simulink model "Poly_creator"  (R2022a)
settings: Solver=FixedStepAuto  StartTime=0.0  StopTime=10  FixedStep=1e-3  RelTol=1e-3
values from neighbouring files (symbols this model uses):
  A = 0.0001    [parameterSetting.m]
  base = Mb*9.8/4+mw*9.8+0.5*9.8*Mv    (= 46305)    [parameterSetting.m]
  d = 2.6    [parameterSetting.m]
  I = 3055e-8    (= 3.055e-05)    [parameterSetting.m]
  Jv = 3044    [parameterSetting.m]
  kf = 6.67e7    (= 66700000)    [parameterSetting.m]
  Kv = 1.5e6    (= 1500000)    [parameterSetting.m]
  L = beamLength(k)*210    [BuildDataSample.m]    (also in parameterSetting.m = 210)
  m = 7800*(7684e-6)    (= 59.9352)    [parameterSetting.m]
  Mv = 4060/2    (= 2030)    [parameterSetting.m]
  mw = 1670/2    (= 835)    [parameterSetting.m]
  N1 = 0    [DatacreatorForChapter4.m]
  N2 = 0    [DatacreatorForChapter4.m]
  position = 0.55*L    [DatacreatorForChapter4.m]    (also in Figure4_9.m = 94.5; chapter4_3.m = 105; parameterSetting.m = 105)
  r = 0.25    [chapter4_3.m]    (also in parameterSetting.m = 0.42)
  v = vkmh/3.6    [BuildDataSample.m]    (also in DatacreatorForChapter4.m = vkmh_set(j)/3.6; Figure4_9.m = vkmh_set(i); chapter4_1.m = vkmh_set(i)/3.6; chapter4_3.m = 13.8888888889)
(part 2 of 79)

== system Poly_creator/Subsystem3/Subsystem1/Subsystem4 ==
  In1 : Inport
  In2 : Inport  Port=2
  Add : Sum  Inputs=++++++++++++++++++++
  Add Constant{,1..18} (19 alike) : Bias  Bias=1  SaturateOnIntegerOverflow=off
  Subsystem1 : Subsystem
  Subsystem10 : Subsystem
  Subsystem11 : Subsystem
  Subsystem12 : Subsystem
  Subsystem13 : Subsystem
  Subsystem14 : Subsystem
  Subsystem15 : Subsystem
  Subsystem16 : Subsystem
  Subsystem17 : Subsystem
  Subsystem18 : Subsystem
  Subsystem19 : Subsystem
  Subsystem2 : Subsystem
  Subsystem20 : Subsystem
  Subsystem3 : Subsystem
  Subsystem4 : Subsystem
  Subsystem5 : Subsystem
  Subsystem6 : Subsystem
  Subsystem7 : Subsystem
  Subsystem8 : Subsystem
  Subsystem9 : Subsystem
  Out1 : Outport
  wires:
  Add Constant7/1 -> Subsystem13/1(index), Add Constant8/1
  Subsystem15/1(Out1) -> Add/15
  [Ff] -> Subsystem3/2(F)
  Add Constant14/1 -> Subsystem12/1(index), Add Constant15/1
  Subsystem8/1(Out1) -> Add/5
  [Ff] -> Subsystem7/2(F)
  Add Constant8/1 -> Subsystem9/1(index), Add Constant9/1
  Subsystem9/1(Out1) -> Add/10
  [Ff] -> Subsystem6/2(F)
  Add Constant4/1 -> Subsystem4/1(index), Add Constant5/1
  Subsystem18/1(Out1) -> Add/20
  Add Constant15/1 -> Subsystem15/1(index), Add Constant16/1
  Subsystem4/1(Out1) -> Add/6
  Add Constant6/1 -> Subsystem6/1(index), Add Constant7/1
  Subsystem16/1(Out1) -> Add/13
  In1/1 -> Add Constant/1, Subsystem5/1(index)
  Subsystem20/1(Out1) -> Add/17
  [Ff] -> Subsystem17/2(F)
  [Ff] -> Subsystem10/2(F)
  Add Constant10/1 -> Subsystem10/1(index), Add Constant11/1
  Add Constant12/1 -> Subsystem17/1(index), Add Constant13/1
  Add Constant3/1 -> Subsystem8/1(index), Add Constant4/1
  Add Constant/1 -> Subsystem1/1(index), Add Constant1/1
  [Ff] -> Subsystem11/2(F)
  Subsystem11/1(Out1) -> Add/11
  [Ff] -> Subsystem19/2(F)
  Subsystem5/1(Out1) -> Add/1
  [Ff] -> Subsystem15/2(F)
  Subsystem1/1(Out1) -> Add/2
  Add Constant13/1 -> Subsystem19/1(index), Add Constant18/1
  [Ff] -> Subsystem9/2(F)
  Add Constant1/1 -> Subsystem3/1(index), Add Constant2/1
  Subsystem10/1(Out1) -> Add/12
  Add Constant2/1 -> Subsystem2/1(index), Add Constant3/1
  [Ff] -> Subsystem2/2(F)
  Subsystem12/1(Out1) -> Add/14
  [Ff] -> Subsystem18/2(F)
  [Ff] -> Subsystem4/2(F)
  Subsystem17/1(Out1) -> Add/18
  Subsystem13/1(Out1) -> Add/9
  [Ff] -> Subsystem1/2(F)
  [Ff] -> Subsystem16/2(F)
  Subsystem14/1(Out1) -> Add/16
  [Ff] -> Subsystem5/2(F)
  [Ff] -> Subsystem20/2(F)
  Subsystem3/1(Out1) -> Add/3
  Subsystem7/1(Out1) -> Add/7
  Add/1 -> Out1/1
  [Ff] -> Subsystem14/2(F)
  [Ff] -> Subsystem8/2(F)
  Subsystem19/1(Out1) -> Add/19
  [Ff] -> Subsystem12/2(F)
  [Ff] -> Subsystem13/2(F)
  Add Constant9/1 -> Subsystem11/1(index), Add Constant10/1
  Add Constant5/1 -> Subsystem7/1(index), Add Constant6/1
  Subsystem6/1(Out1) -> Add/8
  Add Constant11/1 -> Subsystem16/1(index), Add Constant14/1
  Subsystem2/1(Out1) -> Add/4
  Add Constant16/1 -> Subsystem14/1(index), Add Constant17/1
  Add Constant17/1 -> Subsystem20/1(index), Add Constant12/1
  Add Constant18/1 -> Subsystem18/1(index)
  In2/1 -> [Ff]

== system Poly_creator/Subsystem3/Subsystem1/Subsystem5 ==
  In1 : Inport
  In2 : Inport  Port=2
  Add : Sum  Inputs=++++++++++++++++++++
  Add Constant{,1..18} (19 alike) : Bias  Bias=1  SaturateOnIntegerOverflow=off
  Subsystem1 : Subsystem
  Subsystem10 : Subsystem
  Subsystem11 : Subsystem
  Subsystem12 : Subsystem
  Subsystem13 : Subsystem
  Subsystem14 : Subsystem
  Subsystem15 : Subsystem
  Subsystem16 : Subsystem
  Subsystem17 : Subsystem
  Subsystem18 : Subsystem
  Subsystem19 : Subsystem
  Subsystem2 : Subsystem
  Subsystem20 : Subsystem
  Subsystem3 : Subsystem
  Subsystem4 : Subsystem
  Subsystem5 : Subsystem
  Subsystem6 : Subsystem
  Subsystem7 : Subsystem
  Subsystem8 : Subsystem
  Subsystem9 : Subsystem
  Out1 : Outport
  wires:
  In2/1 -> [Ff]
  Add Constant18/1 -> Subsystem18/1(index)
  Add Constant17/1 -> Add Constant12/1, Subsystem20/1(index)
  Add Constant16/1 -> Add Constant17/1, Subsystem14/1(index)
  Subsystem2/1(Out1) -> Add/4
  Add Constant11/1 -> Add Constant14/1, Subsystem16/1(index)
  Subsystem6/1(Out1) -> Add/8
  Add Constant5/1 -> Add Constant6/1, Subsystem7/1(index)
  Add Constant9/1 -> Add Constant10/1, Subsystem11/1(index)
  [Ff] -> Subsystem13/2(F)
  [Ff] -> Subsystem12/2(F)
  Subsystem19/1(Out1) -> Add/19
  [Ff] -> Subsystem8/2(F)
  [Ff] -> Subsystem14/2(F)
  Add/1 -> Out1/1
  Subsystem7/1(Out1) -> Add/7
  Subsystem3/1(Out1) -> Add/3
  [Ff] -> Subsystem20/2(F)
  [Ff] -> Subsystem5/2(F)
  Subsystem14/1(Out1) -> Add/16
  [Ff] -> Subsystem16/2(F)
  [Ff] -> Subsystem1/2(F)
  Subsystem13/1(Out1) -> Add/9
  Subsystem17/1(Out1) -> Add/18
  [Ff] -> Subsystem4/2(F)
  [Ff] -> Subsystem18/2(F)
  Subsystem12/1(Out1) -> Add/14
  [Ff] -> Subsystem2/2(F)
  Add Constant2/1 -> Add Constant3/1, Subsystem2/1(index)
  Subsystem10/1(Out1) -> Add/12
  Add Constant1/1 -> Add Constant2/1, Subsystem3/1(index)
  [Ff] -> Subsystem9/2(F)
  Add Constant13/1 -> Add Constant18/1, Subsystem19/1(index)
  Subsystem1/1(Out1) -> Add/2
  [Ff] -> Subsystem15/2(F)
  Subsystem5/1(Out1) -> Add/1
  [Ff] -> Subsystem19/2(F)
  Subsystem11/1(Out1) -> Add/11
  [Ff] -> Subsystem11/2(F)
  Add Constant/1 -> Add Constant1/1, Subsystem1/1(index)
  Add Constant3/1 -> Add Constant4/1, Subsystem8/1(index)
  Add Constant12/1 -> Add Constant13/1, Subsystem17/1(index)
  Add Constant10/1 -> Add Constant11/1, Subsystem10/1(index)
  [Ff] -> Subsystem10/2(F)
  [Ff] -> Subsystem17/2(F)
  Subsystem20/1(Out1) -> Add/17
  In1/1 -> Subsystem5/1(index), Add Constant/1
  Subsystem16/1(Out1) -> Add/13
  Add Constant6/1 -> Add Constant7/1, Subsystem6/1(index)
  Subsystem4/1(Out1) -> Add/6
  Add Constant15/1 -> Add Constant16/1, Subsystem15/1(index)
  Subsystem18/1(Out1) -> Add/20
  Add Constant4/1 -> Add Constant5/1, Subsystem4/1(index)
  [Ff] -> Subsystem6/2(F)
  Subsystem9/1(Out1) -> Add/10
  Add Constant8/1 -> Add Constant9/1, Subsystem9/1(index)
  [Ff] -> Subsystem7/2(F)
  Subsystem8/1(Out1) -> Add/5
  Add Constant14/1 -> Add Constant15/1, Subsystem12/1(index)
  [Ff] -> Subsystem3/2(F)
  Subsystem15/1(Out1) -> Add/15
  Add Constant7/1 -> Add Constant8/1, Subsystem13/1(index)

== system Poly_creator/Subsystem3/Subsystem1/Subsystem6 ==
  In1 : Inport
  In2 : Inport  Port=2
  Add : Sum  Inputs=++++++++++++++++++++
  Add Constant{,1..18} (19 alike) : Bias  Bias=1  SaturateOnIntegerOverflow=off
  Subsystem1 : Subsystem
  Subsystem10 : Subsystem
  Subsystem11 : Subsystem
  Subsystem12 : Subsystem
  Subsystem13 : Subsystem
  Subsystem14 : Subsystem
  Subsystem15 : Subsystem
  Subsystem16 : Subsystem
  Subsystem17 : Subsystem
  Subsystem18 : Subsystem
  Subsystem19 : Subsystem
  Subsystem2 : Subsystem
  Subsystem20 : Subsystem
  Subsystem3 : Subsystem
  Subsystem4 : Subsystem
  Subsystem5 : Subsystem
  Subsystem6 : Subsystem
  Subsystem7 : Subsystem
  Subsystem8 : Subsystem
  Subsystem9 : Subsystem
  Out1 : Outport
  wires:
  Add Constant7/1 -> Subsystem13/1(index), Add Constant8/1
  Subsystem15/1(Out1) -> Add/15
  [Ff] -> Subsystem3/2(F)
  Add Constant14/1 -> Subsystem12/1(index), Add Constant15/1
  Subsystem8/1(Out1) -> Add/5
  [Ff] -> Subsystem7/2(F)
  Add Constant8/1 -> Subsystem9/1(index), Add Constant9/1
  Subsystem9/1(Out1) -> Add/10
  [Ff] -> Subsystem6/2(F)
  Add Constant4/1 -> Subsystem4/1(index), Add Constant5/1
  Subsystem18/1(Out1) -> Add/20
  Add Constant15/1 -> Subsystem15/1(index), Add Constant16/1
  Subsystem4/1(Out1) -> Add/6
  Add Constant6/1 -> Subsystem6/1(index), Add Constant7/1
  Subsystem16/1(Out1) -> Add/13
  In1/1 -> Add Constant/1, Subsystem5/1(index)
  Subsystem20/1(Out1) -> Add/17
  [Ff] -> Subsystem17/2(F)
  [Ff] -> Subsystem10/2(F)
  Add Constant10/1 -> Subsystem10/1(index), Add Constant11/1
  Add Constant12/1 -> Subsystem17/1(index), Add Constant13/1
  Add Constant3/1 -> Subsystem8/1(index), Add Constant4/1
  Add Constant/1 -> Subsystem1/1(index), Add Constant1/1
  [Ff] -> Subsystem11/2(F)
  Subsystem11/1(Out1) -> Add/11
  [Ff] -> Subsystem19/2(F)
  Subsystem5/1(Out1) -> Add/1
  [Ff] -> Subsystem15/2(F)
  Subsystem1/1(Out1) -> Add/2
  Add Constant13/1 -> Subsystem19/1(index), Add Constant18/1
  [Ff] -> Subsystem9/2(F)
  Add Constant1/1 -> Subsystem3/1(index), Add Constant2/1
  Subsystem10/1(Out1) -> Add/12
  Add Constant2/1 -> Subsystem2/1(index), Add Constant3/1
  [Ff] -> Subsystem2/2(F)
  Subsystem12/1(Out1) -> Add/14
  [Ff] -> Subsystem18/2(F)
  [Ff] -> Subsystem4/2(F)
  Subsystem17/1(Out1) -> Add/18
  Subsystem13/1(Out1) -> Add/9
  [Ff] -> Subsystem1/2(F)
  [Ff] -> Subsystem16/2(F)
  Subsystem14/1(Out1) -> Add/16
  [Ff] -> Subsystem5/2(F)
  [Ff] -> Subsystem20/2(F)
  Subsystem3/1(Out1) -> Add/3
  Subsystem7/1(Out1) -> Add/7
  Add/1 -> Out1/1
  [Ff] -> Subsystem14/2(F)
  [Ff] -> Subsystem8/2(F)
  Subsystem19/1(Out1) -> Add/19
  [Ff] -> Subsystem12/2(F)
  [Ff] -> Subsystem13/2(F)
  Add Constant9/1 -> Subsystem11/1(index), Add Constant10/1
  Add Constant5/1 -> Subsystem7/1(index), Add Constant6/1
  Subsystem6/1(Out1) -> Add/8
  Add Constant11/1 -> Subsystem16/1(index), Add Constant14/1
  Subsystem2/1(Out1) -> Add/4
  Add Constant16/1 -> Subsystem14/1(index), Add Constant17/1
  Add Constant17/1 -> Subsystem20/1(index), Add Constant12/1
  Add Constant18/1 -> Subsystem18/1(index)
  In2/1 -> [Ff]

== system Poly_creator/Subsystem3/Subsystem3/Subsystem1 ==
  In2 : Inport
  In1 : Inport  Port=2
  Add : Sum  Inputs=++++++++++++++++++++
  Add Constant{,1..16,19..20} (19 alike) : Bias  Bias=1  SaturateOnIntegerOverflow=off
  Subsystem1 : Subsystem
  Subsystem10 : Subsystem
  Subsystem11 : Subsystem
  Subsystem12 : Subsystem
  Subsystem13 : Subsystem
  Subsystem14 : Subsystem
  Subsystem15 : Subsystem
  Subsystem16 : Subsystem
  Subsystem17 : Subsystem
  Subsystem18 : Subsystem
  Subsystem19 : Subsystem
  Subsystem2 : Subsystem
  Subsystem21 : Subsystem
  Subsystem3 : Subsystem
  Subsystem4 : Subsystem
  Subsystem5 : Subsystem
  Subsystem6 : Subsystem
  Subsystem7 : Subsystem
  Subsystem8 : Subsystem
  Subsystem9 : Subsystem
  Out1 : Outport
  wires:
  [Ff] -> Subsystem19/2(F)
  Subsystem16/1(Out1) -> Add/18
  Add Constant13/1 -> Subsystem16/1(index), Add Constant19/1
  Add Constant3/1 -> Add Constant4/1, Subsystem6/1(index)
  Add Constant9/1 -> Add Constant10/1, Subsystem11/1(index)
  Add Constant19/1 -> Subsystem19/1(index), Add Constant20/1
  [Ff] -> Subsystem14/2(F)
  [Ff] -> Subsystem5/2(F)
  Add Constant16/1 -> Add Constant12/1, Subsystem13/1(index)
  Add Constant2/1 -> Add Constant3/1, Subsystem2/1(index)
  [Ff] -> Subsystem2/2(F)
  [Ff] -> Subsystem11/2(F)
  Add Constant/1 -> Add Constant1/1, Subsystem1/1(index)
  Add Constant6/1 -> Add Constant7/1, Subsystem5/1(index)
  [Ff] -> Subsystem7/2(F)
  Add Constant14/1 -> Add Constant15/1, Subsystem12/1(index)
  [Ff] -> Subsystem9/2(F)
  [Ff] -> Subsystem8/2(F)
  [Ff] -> Subsystem16/2(F)
  Add Constant15/1 -> Add Constant16/1, Subsystem15/1(index)
  [Ff] -> Subsystem4/2(F)
  Add Constant20/1 -> Subsystem17/1(index)
  [Ff] -> Subsystem1/2(F)
  [Ff] -> Subsystem18/2(F)
  Add Constant4/1 -> Add Constant5/1, Subsystem4/1(index)
  [Ff] -> Subsystem21/2(F)
  Add Constant5/1 -> Add Constant6/1, Subsystem7/1(index)
  Add Constant12/1 -> Subsystem18/1(index), Add Constant13/1
  In1/1 -> Add Constant/1, Subsystem21/1(index)
  [Ff] -> Subsystem15/2(F)
  Add Constant11/1 -> Add Constant14/1, Subsystem14/1(index)
  Add/1 -> Out1/1
  [Ff] -> Subsystem17/2(F)
  Add Constant1/1 -> Add Constant2/1, Subsystem3/1(index)
  Add Constant8/1 -> Add Constant9/1, Subsystem8/1(index)
  [Ff] -> Subsystem12/2(F)
  Add Constant10/1 -> Add Constant11/1, Subsystem9/1(index)
  [Ff] -> Subsystem6/2(F)
  Add Constant7/1 -> Add Constant8/1, Subsystem10/1(index)
  [Ff] -> Subsystem10/2(F)
  [Ff] -> Subsystem13/2(F)
  [Ff] -> Subsystem3/2(F)
  In2/1 -> [Ff]
  Subsystem19/1(Out1) -> Add/19
  Subsystem17/1(Out1) -> Add/20
  Subsystem18/1(Out1) -> Add/17
  Subsystem13/1(Out1) -> Add/16
  Subsystem15/1(Out1) -> Add/15
  Subsystem10/1(Out1) -> Add/9
  Subsystem8/1(Out1) -> Add/10
  Subsystem11/1(Out1) -> Add/11
  Subsystem12/1(Out1) -> Add/14
  Subsystem14/1(Out1) -> Add/13
  Subsystem9/1(Out1) -> Add/12
  Subsystem21/1(Out1) -> Add/1
  Subsystem1/1(Out1) -> Add/2
  Subsystem3/1(Out1) -> Add/3
  Subsystem2/1(Out1) -> Add/4
  Subsystem6/1(Out1) -> Add/5
  Subsystem5/1(Out1) -> Add/8
  Subsystem7/1(Out1) -> Add/7
  Subsystem4/1(Out1) -> Add/6

== system Poly_creator/Subsystem3/Subsystem3/Subsystem2 ==
  In2 : Inport
  In1 : Inport  Port=2
  Add : Sum  Inputs=++++++++++++++++++++
  Add Constant{,1..16,19..20} (19 alike) : Bias  Bias=1  SaturateOnIntegerOverflow=off
  Subsystem1 : Subsystem
  Subsystem10 : Subsystem
  Subsystem11 : Subsystem
  Subsystem12 : Subsystem
  Subsystem13 : Subsystem
  Subsystem14 : Subsystem
  Subsystem15 : Subsystem
  Subsystem16 : Subsystem
  Subsystem17 : Subsystem
  Subsystem18 : Subsystem
  Subsystem19 : Subsystem
  Subsystem2 : Subsystem
  Subsystem21 : Subsystem
  Subsystem3 : Subsystem
  Subsystem4 : Subsystem
  Subsystem5 : Subsystem
  Subsystem6 : Subsystem
  Subsystem7 : Subsystem
  Subsystem8 : Subsystem
  Subsystem9 : Subsystem
  Out1 : Outport
  wires:
  Subsystem4/1(Out1) -> Add/6
  Subsystem7/1(Out1) -> Add/7
  Subsystem5/1(Out1) -> Add/8
  Subsystem6/1(Out1) -> Add/5
  Subsystem2/1(Out1) -> Add/4
  Subsystem3/1(Out1) -> Add/3
  Subsystem1/1(Out1) -> Add/2
  Subsystem21/1(Out1) -> Add/1
  Subsystem9/1(Out1) -> Add/12
  Subsystem14/1(Out1) -> Add/13
  Subsystem12/1(Out1) -> Add/14
  Subsystem11/1(Out1) -> Add/11
  Subsystem8/1(Out1) -> Add/10
  Subsystem10/1(Out1) -> Add/9
  Subsystem15/1(Out1) -> Add/15
  Subsystem13/1(Out1) -> Add/16
  Subsystem18/1(Out1) -> Add/17
  Subsystem17/1(Out1) -> Add/20
  Subsystem19/1(Out1) -> Add/19
  In2/1 -> [Ff]
  [Ff] -> Subsystem3/2(F)
  [Ff] -> Subsystem13/2(F)
  [Ff] -> Subsystem10/2(F)
  Add Constant7/1 -> Subsystem10/1(index), Add Constant8/1
  [Ff] -> Subsystem6/2(F)
  Add Constant10/1 -> Subsystem9/1(index), Add Constant11/1
  [Ff] -> Subsystem12/2(F)
  Add Constant8/1 -> Subsystem8/1(index), Add Constant9/1
  Add Constant1/1 -> Subsystem3/1(index), Add Constant2/1
  [Ff] -> Subsystem17/2(F)
  Add/1 -> Out1/1
  Add Constant11/1 -> Subsystem14/1(index), Add Constant14/1
  [Ff] -> Subsystem15/2(F)
  In1/1 -> Subsystem21/1(index), Add Constant/1
  Add Constant12/1 -> Add Constant13/1, Subsystem18/1(index)
  Add Constant5/1 -> Subsystem7/1(index), Add Constant6/1
  [Ff] -> Subsystem21/2(F)
  Add Constant4/1 -> Subsystem4/1(index), Add Constant5/1
  [Ff] -> Subsystem18/2(F)
  [Ff] -> Subsystem1/2(F)
  Add Constant20/1 -> Subsystem17/1(index)
  [Ff] -> Subsystem4/2(F)
  Add Constant15/1 -> Subsystem15/1(index), Add Constant16/1
  [Ff] -> Subsystem16/2(F)
  [Ff] -> Subsystem8/2(F)
  [Ff] -> Subsystem9/2(F)
  Add Constant14/1 -> Subsystem12/1(index), Add Constant15/1
  [Ff] -> Subsystem7/2(F)
  Add Constant6/1 -> Subsystem5/1(index), Add Constant7/1
  Add Constant/1 -> Subsystem1/1(index), Add Constant1/1
  [Ff] -> Subsystem11/2(F)
  [Ff] -> Subsystem2/2(F)
  Add Constant2/1 -> Subsystem2/1(index), Add Constant3/1
  Add Constant16/1 -> Subsystem13/1(index), Add Constant12/1
  [Ff] -> Subsystem5/2(F)
  [Ff] -> Subsystem14/2(F)
  Add Constant19/1 -> Add Constant20/1, Subsystem19/1(index)
  Add Constant9/1 -> Subsystem11/1(index), Add Constant10/1
  Add Constant3/1 -> Subsystem6/1(index), Add Constant4/1
  Add Constant13/1 -> Add Constant19/1, Subsystem16/1(index)
  Subsystem16/1(Out1) -> Add/18
  [Ff] -> Subsystem19/2(F)

== system Poly_creator/Subsystem3/Subsystem3/Subsystem3 ==
  In2 : Inport
  In1 : Inport  Port=2
  Add : Sum  Inputs=++++++++++++++++++++
  Add Constant{,1..16,19..20} (19 alike) : Bias  Bias=1  SaturateOnIntegerOverflow=off
  Subsystem1 : Subsystem
  Subsystem10 : Subsystem
  Subsystem11 : Subsystem
  Subsystem12 : Subsystem
  Subsystem13 : Subsystem
  Subsystem14 : Subsystem
  Subsystem15 : Subsystem
  Subsystem16 : Subsystem
  Subsystem17 : Subsystem
  Subsystem18 : Subsystem
  Subsystem19 : Subsystem
  Subsystem2 : Subsystem
  Subsystem21 : Subsystem
  Subsystem3 : Subsystem
  Subsystem4 : Subsystem
  Subsystem5 : Subsystem
  Subsystem6 : Subsystem
  Subsystem7 : Subsystem
  Subsystem8 : Subsystem
  Subsystem9 : Subsystem
  Out1 : Outport
  wires:
  [Ff] -> Subsystem19/2(F)
  Subsystem16/1(Out1) -> Add/18
  Add Constant13/1 -> Subsystem16/1(index), Add Constant19/1
  Add Constant3/1 -> Add Constant4/1, Subsystem6/1(index)
  Add Constant9/1 -> Add Constant10/1, Subsystem11/1(index)
  Add Constant19/1 -> Subsystem19/1(index), Add Constant20/1
  [Ff] -> Subsystem14/2(F)
  [Ff] -> Subsystem5/2(F)
  Add Constant16/1 -> Add Constant12/1, Subsystem13/1(index)
  Add Constant2/1 -> Add Constant3/1, Subsystem2/1(index)
  [Ff] -> Subsystem2/2(F)
  [Ff] -> Subsystem11/2(F)
  Add Constant/1 -> Add Constant1/1, Subsystem1/1(index)
  Add Constant6/1 -> Add Constant7/1, Subsystem5/1(index)
  [Ff] -> Subsystem7/2(F)
  Add Constant14/1 -> Add Constant15/1, Subsystem12/1(index)
  [Ff] -> Subsystem9/2(F)
  [Ff] -> Subsystem8/2(F)
  [Ff] -> Subsystem16/2(F)
  Add Constant15/1 -> Add Constant16/1, Subsystem15/1(index)
  [Ff] -> Subsystem4/2(F)
  Add Constant20/1 -> Subsystem17/1(index)
  [Ff] -> Subsystem1/2(F)
  [Ff] -> Subsystem18/2(F)
  Add Constant4/1 -> Add Constant5/1, Subsystem4/1(index)
  [Ff] -> Subsystem21/2(F)
  Add Constant5/1 -> Add Constant6/1, Subsystem7/1(index)
  Add Constant12/1 -> Subsystem18/1(index), Add Constant13/1
  In1/1 -> Add Constant/1, Subsystem21/1(index)
  [Ff] -> Subsystem15/2(F)
  Add Constant11/1 -> Add Constant14/1, Subsystem14/1(index)
  Add/1 -> Out1/1
  [Ff] -> Subsystem17/2(F)
  Add Constant1/1 -> Add Constant2/1, Subsystem3/1(index)
  Add Constant8/1 -> Add Constant9/1, Subsystem8/1(index)
  [Ff] -> Subsystem12/2(F)
  Add Constant10/1 -> Add Constant11/1, Subsystem9/1(index)
  [Ff] -> Subsystem6/2(F)
  Add Constant7/1 -> Add Constant8/1, Subsystem10/1(index)
  [Ff] -> Subsystem10/2(F)
  [Ff] -> Subsystem13/2(F)
  [Ff] -> Subsystem3/2(F)
  In2/1 -> [Ff]
  Subsystem19/1(Out1) -> Add/19
  Subsystem17/1(Out1) -> Add/20
  Subsystem18/1(Out1) -> Add/17
  Subsystem13/1(Out1) -> Add/16
  Subsystem15/1(Out1) -> Add/15
  Subsystem10/1(Out1) -> Add/9
  Subsystem8/1(Out1) -> Add/10
  Subsystem11/1(Out1) -> Add/11
  Subsystem12/1(Out1) -> Add/14
  Subsystem14/1(Out1) -> Add/13
  Subsystem9/1(Out1) -> Add/12
  Subsystem21/1(Out1) -> Add/1
  Subsystem1/1(Out1) -> Add/2
  Subsystem3/1(Out1) -> Add/3
  Subsystem2/1(Out1) -> Add/4
  Subsystem6/1(Out1) -> Add/5
  Subsystem5/1(Out1) -> Add/8
  Subsystem7/1(Out1) -> Add/7
  Subsystem4/1(Out1) -> Add/6

== system Poly_creator/Subsystem3/Subsystem3/Subsystem4 ==
  In2 : Inport
  In1 : Inport  Port=2
  Add : Sum  Inputs=++++++++++++++++++++
  Add Constant{,1..16,19..20} (19 alike) : Bias  Bias=1  SaturateOnIntegerOverflow=off
  Subsystem1 : Subsystem
  Subsystem10 : Subsystem
  Subsystem11 : Subsystem
  Subsystem12 : Subsystem
  Subsystem13 : Subsystem
  Subsystem14 : Subsystem
  Subsystem15 : Subsystem
  Subsystem16 : Subsystem
  Subsystem17 : Subsystem
  Subsystem18 : Subsystem
  Subsystem19 : Subsystem
  Subsystem2 : Subsystem
  Subsystem21 : Subsystem
  Subsystem3 : Subsystem
  Subsystem4 : Subsystem
  Subsystem5 : Subsystem
  Subsystem6 : Subsystem
  Subsystem7 : Subsystem
  Subsystem8 : Subsystem
  Subsystem9 : Subsystem
  Out1 : Outport
  wires:
  Subsystem4/1(Out1) -> Add/6
  Subsystem7/1(Out1) -> Add/7
  Subsystem5/1(Out1) -> Add/8
  Subsystem6/1(Out1) -> Add/5
  Subsystem2/1(Out1) -> Add/4
  Subsystem3/1(Out1) -> Add/3
  Subsystem1/1(Out1) -> Add/2
  Subsystem21/1(Out1) -> Add/1
  Subsystem9/1(Out1) -> Add/12
  Subsystem14/1(Out1) -> Add/13
  Subsystem12/1(Out1) -> Add/14
  Subsystem11/1(Out1) -> Add/11
  Subsystem8/1(Out1) -> Add/10
  Subsystem10/1(Out1) -> Add/9
  Subsystem15/1(Out1) -> Add/15
  Subsystem13/1(Out1) -> Add/16
  Subsystem18/1(Out1) -> Add/17
  Subsystem17/1(Out1) -> Add/20
  Subsystem19/1(Out1) -> Add/19
  In2/1 -> [Ff]
  [Ff] -> Subsystem3/2(F)
  [Ff] -> Subsystem13/2(F)
  [Ff] -> Subsystem10/2(F)
  Add Constant7/1 -> Subsystem10/1(index), Add Constant8/1
  [Ff] -> Subsystem6/2(F)
  Add Constant10/1 -> Subsystem9/1(index), Add Constant11/1
  [Ff] -> Subsystem12/2(F)
  Add Constant8/1 -> Subsystem8/1(index), Add Constant9/1
  Add Constant1/1 -> Subsystem3/1(index), Add Constant2/1
  [Ff] -> Subsystem17/2(F)
  Add/1 -> Out1/1
  Add Constant11/1 -> Subsystem14/1(index), Add Constant14/1
  [Ff] -> Subsystem15/2(F)
  In1/1 -> Subsystem21/1(index), Add Constant/1
  Add Constant12/1 -> Add Constant13/1, Subsystem18/1(index)
  Add Constant5/1 -> Subsystem7/1(index), Add Constant6/1
  [Ff] -> Subsystem21/2(F)
  Add Constant4/1 -> Subsystem4/1(index), Add Constant5/1
  [Ff] -> Subsystem18/2(F)
  [Ff] -> Subsystem1/2(F)
  Add Constant20/1 -> Subsystem17/1(index)
  [Ff] -> Subsystem4/2(F)
  Add Constant15/1 -> Subsystem15/1(index), Add Constant16/1
  [Ff] -> Subsystem16/2(F)
  [Ff] -> Subsystem8/2(F)
  [Ff] -> Subsystem9/2(F)
  Add Constant14/1 -> Subsystem12/1(index), Add Constant15/1
  [Ff] -> Subsystem7/2(F)
  Add Constant6/1 -> Subsystem5/1(index), Add Constant7/1
  Add Constant/1 -> Subsystem1/1(index), Add Constant1/1
  [Ff] -> Subsystem11/2(F)
  [Ff] -> Subsystem2/2(F)
  Add Constant2/1 -> Subsystem2/1(index), Add Constant3/1
  Add Constant16/1 -> Subsystem13/1(index), Add Constant12/1
  [Ff] -> Subsystem5/2(F)
  [Ff] -> Subsystem14/2(F)
  Add Constant19/1 -> Add Constant20/1, Subsystem19/1(index)
  Add Constant9/1 -> Subsystem11/1(index), Add Constant10/1
  Add Constant3/1 -> Subsystem6/1(index), Add Constant4/1
  Add Constant13/1 -> Add Constant19/1, Subsystem16/1(index)
  Subsystem16/1(Out1) -> Add/18
  [Ff] -> Subsystem19/2(F)
